simulink model "ofdm_txrx_mimo_2x"  (Simulink 6.6)
settings: Solver=VariableStepDiscrete  StartTime=0.0  StopTime=20e3  RelTol=1e-3
PreLoadFcn:
  | ofdm_rx_mimo_init
InitFcn:
  | ofdm_rx_mimo_init
StartFcn:
  | ofdm_rx_mimo_init
values from neighbouring files (symbols this model uses):
  BER_RAM_init_size = 2048    [ofdm_tx_mimo_init.m]
  CPLength = 16    [ofdm_tx_mimo_init.m]
  CRCPolynomial = hex2dec('04c11db7')    [ofdm_tx_mimo_init.m]
  freqOffset_track_filtCoef_i = hex2dec('2500')/2^32    [ofdm_rx_mimo_init.m]
  freqOffset_track_filtCoef_p = hex2dec('d0000')/2^32    [ofdm_rx_mimo_init.m]
  hi = zeros(1,length(cci))    [ofdm_rx_mimo_init.m]
  hr = zeros(1,length(ccr))    [ofdm_rx_mimo_init.m]
  max_CP_length = 16    [ofdm_tx_mimo_init.m]
  max_num_baseRateSymbols = 31    [ofdm_tx_mimo_init.m]
  max_num_subcarriers = 64    [ofdm_tx_mimo_init.m]
  max_num_trainingSymbols = 15    [ofdm_tx_mimo_init.m]
  max_numBytes = 16384    [ofdm_tx_mimo_init.m]
  max_OFDM_symbols = 2047    [ofdm_tx_mimo_init.m]
  modConstellation_qam16 = .75*[1 3 -1 -3]./3    [ofdm_tx_mimo_init.m]
  modConstellation_qam256 = 0.9375*[3 1 5 7 11 9 13 15 -3 -1 -5 -7 -11 -9 -13 -15]./15    [ofdm_tx_mimo_init.m]
  modConstellation_qam64 = 0.875*[3 1 5 7 -3 -1 -5 -7]./7    [ofdm_tx_mimo_init.m]
  modConstellation_qpsk = [1 -1]./sqrt(2)    [ofdm_tx_mimo_init.m]
  numBaseRateSymbols = 2    [ofdm_tx_mimo_init.m]
  numSubcarriers = 64    [ofdm_tx_mimo_init.m]
  numTrainingSymbols = 2    [ofdm_tx_mimo_init.m]
  pilot1_indicies = 7 + ( (64-7) * 2^16)    (= 3735559)    [ofdm_tx_mimo_init.m]
  pilot2_indicies = 21 + ( (64-21) * 2^16)    (= 2818069)    [ofdm_tx_mimo_init.m]
  pilotValue_neg = hex2dec('8000') + (2^16 * 0)    [ofdm_tx_mimo_init.m]
  pilotValue_pos = hex2dec('7FFF') + (2^16 * 0)    [ofdm_tx_mimo_init.m]
  pkt_crossCorr_thresh = 0.7    [ofdm_rx_mimo_init.m]
  pkt_energy_thresh = 0    [ofdm_rx_mimo_init.m]
  pkt_numPayloadBytes = 1400+42-6    (= 1436)    [ofdm_tx_mimo_init.m]
  pktByteNums = numHeaderBytes +  				byteIndex_numPayloadBytes(1)*2^8 +  				byteIndex_numPayloadBytes(2)*2^16 +  				byteIndex_simpleDynModMasks * 2^24    [ofdm_rx_mimo_init.m]
  PktDet_Delay = 26 + Symbol_Timing_Offset*2^7    (= 1050)    [ofdm_rx_mimo_init.m]
  preamble = 6*[0 shortsyms_10 longsyms_2]    [ofdm_tx_mimo_init.m]
  preamble_I = real(preamble)    [ofdm_tx_mimo_init.m]
  preamble_Q = imag(preamble)    [ofdm_tx_mimo_init.m]
  RAM_init_size = max_numBytes    (= 16384)    [ofdm_tx_mimo_init.m]
  reg_Interrupt_PktBuf_Control = reg_InterruptControl +  	1 * 2^16 +  	0 * 2^24    (= 65656)    [ofdm_rx_mimo_init.m]
  rx_controlBits = 1 * 2  		 + 1 * 4  		 + 0 * 8  		 + 1 * 16 * rx_SISO_Mode  		 + 1 * 32  		 + 1 * 64  		 + 0 * 128  		 + 1 * 256  		 + 0 * 512  		 + 1 * 1024  		 + 0 * 2048  		 + 1 * 4096  		 + 1 * 8192  		 + 0 * 16384  		 + 1 * 65536  		 + 1 * 131072  		 + 0 * 262144  		 + 1 * 524288    [ofdm_rx_mimo_init.m]
  Rx_PhaseNoiseTrack_K = 0.6094    [ofdm_rx_mimo_init.m]
  rx_postEq_scaling = round(rxScaling*2^11) * (1 + 2^16)    [ofdm_rx_mimo_init.m]
  RxDataScrambling_Seq = [40 198 78 63 82 173 102 245 48 111 172 115 147 230 216 93 72 65 62 2 205 242 122 90 128 83 105 97 73 10 5 252]    [ofdm_tx_mimo_init.m]
  simOnly_numSamples = length(preamble)+( (numSubcarriers+CPLength)*(numTrainingSymbols + numBaseRateSymbols + numFullRateSymbols/2) )    [ofdm_tx_mimo_init.m]
  train = [train train]    [ofdm_tx_mimo_init.m]
  tx_controlBits = (antB_preambleShift * 2^4) + (2^0 * tx_SISO_Mode) + 4*0 + 8*1    (= 73)    [ofdm_tx_mimo_init.m]
  TxDataScrambling_Seq = [40 198 78 63 82 173 102 245 48 111 172 115 147 230 216 93 72 65 62 2 205 242 122 90 128 83 105 97 73 10 5 252]    [ofdm_tx_mimo_init.m]
  txReg_symbolCounts = (2^16 * numFullRateSymbols) + (2^8 *numBaseRateSymbols ) + numTrainingSymbols    [ofdm_tx_mimo_init.m]
  TxRx_FFTScaling = bin2dec('010111') + (bin2dec('000101') * 2^6)    [ofdm_tx_mimo_init.m]
undefined symbols (not defined in the file or in its folder): AntA, AntB, BaseRate, CRC_table_gen, gcbh, intfilt, pktTiming_count_payloadStart, pktTiming_symbol_length, xlBlockLoadCallback, xlGetSimulinkPeriod
libraries: xbsIndex_r4 x3973, dspsigops x4, commchan3 x2, commrflib2 x2, dspsrcs4 x1
(part 19 of 38)

== system ofdm_txrx_mimo_2x/OFDM Rx MIMO/Long Corr 4x/C6/FSM ==
  Coef Addr Gen : xbsIndex_r4/Counter  cnt_type=Free Running  cnt_to=Inf  operation=Up  start_count=0  cnt_by_val=1  arith_type=Unsigned  n_bits=ceil(log2(length(h(1:N))))  bin_pt=0  load_pin=off  rst=on  en=off  explicit_period=off  period=1  dbl_ovrd=off  use_behavioral_HDL=off  use_rpm=on  implementation=Fabric  block_type=counter
      settings: xl_area=[2 3 0 3 0 0 0]  block_version=VER_STRING_GOES_HERE
  Constant2 : xbsIndex_r4/Constant  arith_type=Unsigned  const=0  n_bits=ceil(log2(length(h(1:N))/2))+1  bin_pt=0  explicit_period=off  period=2/N  equ=P=C  opselect=C  inp2=PCIN>>17  opr=+  inp1=P  carry=CIN  dbl_ovrd=off  block_type=constant
      settings: block_version=VER_STRING_GOES_HERE
  Constant3 : xbsIndex_r4/Constant  arith_type=Unsigned  const=length(h(1:N))-1  n_bits=ceil(log2(length(h(1:N))))  bin_pt=0  explicit_period=off  period=2/N  equ=P=C  opselect=C  inp2=PCIN>>17  opr=+  inp1=P  carry=CIN  dbl_ovrd=off  block_type=constant
      settings: block_version=VER_STRING_GOES_HERE
  Data Addr Gen : xbsIndex_r4/Counter  cnt_type=Count Limited  cnt_to=length(h(1:N))-1  operation=Up  start_count=0  cnt_by_val=1  arith_type=Unsigned  n_bits=ceil(log2(length(h(1:N))))  bin_pt=0  load_pin=off  rst=off  en=off  explicit_period=on  period=Ts_In/N  dbl_ovrd=off  use_behavioral_HDL=off  use_rpm=on  implementation=Fabric  block_type=counter
      settings: xl_area=[3 3 0 4 0 0 0]  block_version=VER_STRING_GOES_HERE
  Delay1 : xbsIndex_r4/Delay  en=off  latency=1  dbl_ovrd=off  reg_retiming=off  block_type=delay
      settings: infoedit=Hardware notes: A delay line is a chain, each link of which is an SRL16 followed by a flip-flop. If register retiming is enabled, the delay line is a chain of flip-flops.  xl_area=[2 3 0 0 0 0 0]  block_version=VER_STRING_GOES_HERE
  Gateway Out : xbsIndex_r4/Gateway Out  hdl_port=off  timing_constraint=None  locs_specified=off  LOCs={}  block_type=gatewayout
      settings: infoedit="Gateway out block.  Converts Xilinx fixed point inputs into ouputs of type Simulink integer, double, or fixed point.<P><P>Hardware notes:  In hardware these blocks become top level output ports or are discarded, depending on how they are configured."  xl_area=[0 0 0 0 0 0 0]  block_version=VER_STRING_GOES_HERE
  Gateway Out1 : xbsIndex_r4/Gateway Out  hdl_port=off  timing_constraint=None  locs_specified=off  LOCs={}  block_type=gatewayout
      settings: infoedit="Gateway out block.  Converts Xilinx fixed point inputs into ouputs of type Simulink integer, double, or fixed point.<P><P>Hardware notes:  In hardware these blocks become top level output ports or are discarded, depending on how they are configured."  xl_area=[0 0 0 0 0 0 0]  block_version=VER_STRING_GOES_HERE
  Gateway Out3 : xbsIndex_r4/Gateway Out  hdl_port=off  timing_constraint=None  locs_specified=off  LOCs={}  block_type=gatewayout
      settings: infoedit="Gateway out block.  Converts Xilinx fixed point inputs into ouputs of type Simulink integer, double, or fixed point.<P><P>Hardware notes:  In hardware these blocks become top level output ports or are discarded, depending on how they are configured."  xl_area=[0 0 0 0 0 0 0]  block_version=VER_STRING_GOES_HERE
  Long Corr Scope : Scope  NumInputPorts=3
      settings: YMin=-1~-1~-35  YMax=1~1~25  SaveName=ScopeData4
  Relational2 : xbsIndex_r4/Relational  mode=a=b  en=off  latency=1  dbl_ovrd=off  block_type=relational
      settings: xl_area=[1 1 0 2 0 0 0]  block_version=VER_STRING_GOES_HERE
  Relational3 : xbsIndex_r4/Relational  mode=a=b  en=off  latency=1  dbl_ovrd=off  block_type=relational
      settings: xl_area=[1 1 0 2 0 0 0]  block_version=VER_STRING_GOES_HERE
  bc3 : xbsIndex_r4/Convert  arith_type=Unsigned  n_bits=1  bin_pt=0  quantization=Truncate  overflow=Wrap  latency=0  dbl_ovrd=off  pipeline=off  block_type=convert
      settings: xl_area=[0 0 0 0 0 0 0]  block_version=VER_STRING_GOES_HERE
  Data Addr : Outport
  Coef Addr : Outport  Port=2
  load : Outport  Port=3
  wires:
  Gateway Out3/1 -> Long Corr Scope/3
  Gateway Out1/1 -> Long Corr Scope/2
  Gateway Out/1 -> Long Corr Scope/1
  Data Addr Gen/1 -> Gateway Out/1, Data Addr/1, Relational2/1
  Coef Addr Gen/1 -> Gateway Out1/1, Delay1/1
  Relational2/1 -> Coef Addr Gen/1
  Constant2/1 -> Relational2/2
  Delay1/1 -> Relational3/1, Coef Addr/1
  Constant3/1 -> Relational3/2
  Relational3/1 -> Gateway Out3/1, bc3/1
  bc3/1 -> load/1

== system ofdm_txrx_mimo_2x/OFDM Rx MIMO/Long Corr 4x/C7/C1 ==
  xn : Inport
  DAddr : Inport  Port=2
  CAddr : Inport  Port=3
  LD : Inport  Port=4
  Down Sample2 : xbsIndex_r4/Down Sample  sample_ratio=length(coef2)  sample_phase=First Value of Frame  en=off  latency=0  dbl_ovrd=off  block_type=dsamp
      settings: xl_area=[5 5 0 4 0 0 0]  block_version=VER_STRING_GOES_HERE
  MAC : Subsystem
  R : xbsIndex_r4/Addressable Shift Register  infer_latency=off  depth=length(coef2)  initVector=[0]  en=off  dbl_ovrd=off  use_behavioral_HDL=off  use_rpm=off  block_type=addrsr
      settings: infoedit="Delay of  configurable length.  Any element  in the delay     line   can    be   addressed   and   driven    onto   the   output     port.<P><P>Hardware  notes: Implemented  using SRL16s.     If Virtex-4, Virtex-II or Spartan-3 devices are used, multiple SRLC16s are cascaded together."  xl_area=[1 0 0 2 0 0 0]  block_version=VER_STRING_GOES_HERE
  ROM1 : xbsIndex_r4/ROM  depth=length(coef2)  initVector=coef2  distributed_mem=Distributed memory  rst=off  init_reg=0  en=off  latency=1  arith_type=Unsigned  n_bits=1  bin_pt=0  dbl_ovrd=off  optimize=Area  use_rpm=off  block_type=sprom
      settings: xl_area=[1 1 0 1 0 0 0]  block_version=VER_STRING_GOES_HERE
  yn : Outport
  xnz : Outport  Port=2
  wires:
  xn/1 -> R/1
  DAddr/1 -> R/2
  CAddr/1 -> ROM1/1
  R/1 -> xnz/1, MAC/1(a)
  MAC/1(yn) -> Down Sample2/1
  Down Sample2/1 -> yn/1
  ROM1/1 -> MAC/2(coeff)
  LD/1 -> MAC/3(ld)

== system ofdm_txrx_mimo_2x/OFDM Rx MIMO/Long Corr 4x/C7/C10 ==
  xn : Inport
  DAddr : Inport  Port=2
  CAddr : Inport  Port=3
  LD : Inport  Port=4
  Down Sample1 : xbsIndex_r4/Down Sample  sample_ratio=length(coef2)  sample_phase=First Value of Frame  en=off  latency=0  dbl_ovrd=off  block_type=dsamp
      settings: xl_area=[3 3 0 2 0 0 0]  block_version=VER_STRING_GOES_HERE
  Down Sample2 : xbsIndex_r4/Down Sample  sample_ratio=length(coef2)  sample_phase=First Value of Frame  en=off  latency=0  dbl_ovrd=off  block_type=dsamp
      settings: xl_area=[5 5 0 4 0 0 0]  block_version=VER_STRING_GOES_HERE
  MAC : Subsystem
  R : xbsIndex_r4/Addressable Shift Register  infer_latency=off  depth=length(coef2)  initVector=[0]  en=off  dbl_ovrd=off  use_behavioral_HDL=off  use_rpm=off  block_type=addrsr
      settings: infoedit="Delay of  configurable length.  Any element  in the delay     line   can    be   addressed   and   driven    onto   the   output     port.<P><P>Hardware  notes: Implemented  using SRL16s.     If Virtex-4, Virtex-II or Spartan-3 devices are used, multiple SRLC16s are cascaded together."  xl_area=[1 0 0 2 0 0 0]  block_version=VER_STRING_GOES_HERE
  ROM1 : xbsIndex_r4/ROM  depth=length(coef2)  initVector=coef2  distributed_mem=Distributed memory  rst=off  init_reg=0  en=off  latency=1  arith_type=Unsigned  n_bits=1  bin_pt=0  dbl_ovrd=off  optimize=Area  use_rpm=off  block_type=sprom
      settings: xl_area=[1 1 0 1 0 0 0]  block_version=VER_STRING_GOES_HERE
  yn : Outport
  xnz : Outport  Port=2
  wires:
  Down Sample1/1 -> R/1
  xn/1 -> Down Sample1/1
  DAddr/1 -> R/2
  CAddr/1 -> ROM1/1
  R/1 -> xnz/1, MAC/1(a)
  MAC/1(yn) -> Down Sample2/1
  Down Sample2/1 -> yn/1
  ROM1/1 -> MAC/2(coeff)
  LD/1 -> MAC/3(ld)

== system ofdm_txrx_mimo_2x/OFDM Rx MIMO/Long Corr 4x/C7/C11 ==
  xn : Inport
  DAddr : Inport  Port=2
  CAddr : Inport  Port=3
  LD : Inport  Port=4
  Down Sample1 : xbsIndex_r4/Down Sample  sample_ratio=length(coef2)  sample_phase=First Value of Frame  en=off  latency=0  dbl_ovrd=off  block_type=dsamp
      settings: xl_area=[3 3 0 2 0 0 0]  block_version=VER_STRING_GOES_HERE
  Down Sample2 : xbsIndex_r4/Down Sample  sample_ratio=length(coef2)  sample_phase=First Value of Frame  en=off  latency=0  dbl_ovrd=off  block_type=dsamp
      settings: xl_area=[5 5 0 4 0 0 0]  block_version=VER_STRING_GOES_HERE
  MAC : Subsystem
  R : xbsIndex_r4/Addressable Shift Register  infer_latency=off  depth=length(coef2)  initVector=[0]  en=off  dbl_ovrd=off  use_behavioral_HDL=off  use_rpm=off  block_type=addrsr
      settings: infoedit="Delay of  configurable length.  Any element  in the delay     line   can    be   addressed   and   driven    onto   the   output     port.<P><P>Hardware  notes: Implemented  using SRL16s.     If Virtex-4, Virtex-II or Spartan-3 devices are used, multiple SRLC16s are cascaded together."  xl_area=[1 0 0 2 0 0 0]  block_version=VER_STRING_GOES_HERE
  ROM1 : xbsIndex_r4/ROM  depth=length(coef2)  initVector=coef2  distributed_mem=Distributed memory  rst=off  init_reg=0  en=off  latency=1  arith_type=Unsigned  n_bits=1  bin_pt=0  dbl_ovrd=off  optimize=Area  use_rpm=off  block_type=sprom
      settings: xl_area=[1 1 0 1 0 0 0]  block_version=VER_STRING_GOES_HERE
  yn : Outport
  xnz : Outport  Port=2
  wires:
  Down Sample1/1 -> R/1
  xn/1 -> Down Sample1/1
  DAddr/1 -> R/2
  CAddr/1 -> ROM1/1
  R/1 -> xnz/1, MAC/1(a)
  MAC/1(yn) -> Down Sample2/1
  Down Sample2/1 -> yn/1
  ROM1/1 -> MAC/2(coeff)
  LD/1 -> MAC/3(ld)

== system ofdm_txrx_mimo_2x/OFDM Rx MIMO/Long Corr 4x/C7/C12 ==
  xn : Inport
  DAddr : Inport  Port=2
  CAddr : Inport  Port=3
  LD : Inport  Port=4
  Down Sample1 : xbsIndex_r4/Down Sample  sample_ratio=length(coef2)  sample_phase=First Value of Frame  en=off  latency=0  dbl_ovrd=off  block_type=dsamp
      settings: xl_area=[3 3 0 2 0 0 0]  block_version=VER_STRING_GOES_HERE
  Down Sample2 : xbsIndex_r4/Down Sample  sample_ratio=length(coef2)  sample_phase=First Value of Frame  en=off  latency=0  dbl_ovrd=off  block_type=dsamp
      settings: xl_area=[5 5 0 4 0 0 0]  block_version=VER_STRING_GOES_HERE
  MAC : Subsystem
  R : xbsIndex_r4/Addressable Shift Register  infer_latency=off  depth=length(coef2)  initVector=[0]  en=off  dbl_ovrd=off  use_behavioral_HDL=off  use_rpm=off  block_type=addrsr
      settings: infoedit="Delay of  configurable length.  Any element  in the delay     line   can    be   addressed   and   driven    onto   the   output     port.<P><P>Hardware  notes: Implemented  using SRL16s.     If Virtex-4, Virtex-II or Spartan-3 devices are used, multiple SRLC16s are cascaded together."  xl_area=[1 0 0 2 0 0 0]  block_version=VER_STRING_GOES_HERE
  ROM1 : xbsIndex_r4/ROM  depth=length(coef2)  initVector=coef2  distributed_mem=Distributed memory  rst=off  init_reg=0  en=off  latency=1  arith_type=Unsigned  n_bits=1  bin_pt=0  dbl_ovrd=off  optimize=Area  use_rpm=off  block_type=sprom
      settings: xl_area=[1 1 0 1 0 0 0]  block_version=VER_STRING_GOES_HERE
  yn : Outport
  xnz : Outport  Port=2
  wires:
  Down Sample1/1 -> R/1
  xn/1 -> Down Sample1/1
  DAddr/1 -> R/2
  CAddr/1 -> ROM1/1
  R/1 -> xnz/1, MAC/1(a)
  MAC/1(yn) -> Down Sample2/1
  Down Sample2/1 -> yn/1
  ROM1/1 -> MAC/2(coeff)
  LD/1 -> MAC/3(ld)

== system ofdm_txrx_mimo_2x/OFDM Rx MIMO/Long Corr 4x/C7/C13 ==
  xn : Inport
  DAddr : Inport  Port=2
  CAddr : Inport  Port=3
  LD : Inport  Port=4
  Down Sample1 : xbsIndex_r4/Down Sample  sample_ratio=length(coef2)  sample_phase=First Value of Frame  en=off  latency=0  dbl_ovrd=off  block_type=dsamp
      settings: xl_area=[3 3 0 2 0 0 0]  block_version=VER_STRING_GOES_HERE
  Down Sample2 : xbsIndex_r4/Down Sample  sample_ratio=length(coef2)  sample_phase=First Value of Frame  en=off  latency=0  dbl_ovrd=off  block_type=dsamp
      settings: xl_area=[5 5 0 4 0 0 0]  block_version=VER_STRING_GOES_HERE
  MAC : Subsystem
  R : xbsIndex_r4/Addressable Shift Register  infer_latency=off  depth=length(coef2)  initVector=[0]  en=off  dbl_ovrd=off  use_behavioral_HDL=off  use_rpm=off  block_type=addrsr
      settings: infoedit="Delay of  configurable length.  Any element  in the delay     line   can    be   addressed   and   driven    onto   the   output     port.<P><P>Hardware  notes: Implemented  using SRL16s.     If Virtex-4, Virtex-II or Spartan-3 devices are used, multiple SRLC16s are cascaded together."  xl_area=[1 0 0 2 0 0 0]  block_version=VER_STRING_GOES_HERE
  ROM1 : xbsIndex_r4/ROM  depth=length(coef2)  initVector=coef2  distributed_mem=Distributed memory  rst=off  init_reg=0  en=off  latency=1  arith_type=Unsigned  n_bits=1  bin_pt=0  dbl_ovrd=off  optimize=Area  use_rpm=off  block_type=sprom
      settings: xl_area=[1 1 0 1 0 0 0]  block_version=VER_STRING_GOES_HERE
  yn : Outport
  xnz : Outport  Port=2
  wires:
  Down Sample1/1 -> R/1
  xn/1 -> Down Sample1/1
  DAddr/1 -> R/2
  CAddr/1 -> ROM1/1
  R/1 -> xnz/1, MAC/1(a)
  MAC/1(yn) -> Down Sample2/1
  Down Sample2/1 -> yn/1
  ROM1/1 -> MAC/2(coeff)
  LD/1 -> MAC/3(ld)

== system ofdm_txrx_mimo_2x/OFDM Rx MIMO/Long Corr 4x/C7/C14 ==
  xn : Inport
  DAddr : Inport  Port=2
  CAddr : Inport  Port=3
  LD : Inport  Port=4
  Down Sample1 : xbsIndex_r4/Down Sample  sample_ratio=length(coef2)  sample_phase=First Value of Frame  en=off  latency=0  dbl_ovrd=off  block_type=dsamp
      settings: xl_area=[3 3 0 2 0 0 0]  block_version=VER_STRING_GOES_HERE
  Down Sample2 : xbsIndex_r4/Down Sample  sample_ratio=length(coef2)  sample_phase=First Value of Frame  en=off  latency=0  dbl_ovrd=off  block_type=dsamp
      settings: xl_area=[5 5 0 4 0 0 0]  block_version=VER_STRING_GOES_HERE
  MAC : Subsystem
  R : xbsIndex_r4/Addressable Shift Register  infer_latency=off  depth=length(coef2)  initVector=[0]  en=off  dbl_ovrd=off  use_behavioral_HDL=off  use_rpm=off  block_type=addrsr
      settings: infoedit="Delay of  configurable length.  Any element  in the delay     line   can    be   addressed   and   driven    onto   the   output     port.<P><P>Hardware  notes: Implemented  using SRL16s.     If Virtex-4, Virtex-II or Spartan-3 devices are used, multiple SRLC16s are cascaded together."  xl_area=[1 0 0 2 0 0 0]  block_version=VER_STRING_GOES_HERE
  ROM1 : xbsIndex_r4/ROM  depth=length(coef2)  initVector=coef2  distributed_mem=Distributed memory  rst=off  init_reg=0  en=off  latency=1  arith_type=Unsigned  n_bits=1  bin_pt=0  dbl_ovrd=off  optimize=Area  use_rpm=off  block_type=sprom
      settings: xl_area=[1 1 0 1 0 0 0]  block_version=VER_STRING_GOES_HERE
  yn : Outport
  xnz : Outport  Port=2
  wires:
  LD/1 -> MAC/3(ld)
  ROM1/1 -> MAC/2(coeff)
  Down Sample2/1 -> yn/1
  MAC/1(yn) -> Down Sample2/1
  R/1 -> MAC/1(a), xnz/1
  CAddr/1 -> ROM1/1
  DAddr/1 -> R/2
  xn/1 -> Down Sample1/1
  Down Sample1/1 -> R/1

== system ofdm_txrx_mimo_2x/OFDM Rx MIMO/Long Corr 4x/C7/C15 ==
  xn : Inport
  DAddr : Inport  Port=2
  CAddr : Inport  Port=3
  LD : Inport  Port=4
  Down Sample1 : xbsIndex_r4/Down Sample  sample_ratio=length(coef2)  sample_phase=First Value of Frame  en=off  latency=0  dbl_ovrd=off  block_type=dsamp
      settings: xl_area=[3 3 0 2 0 0 0]  block_version=VER_STRING_GOES_HERE
  Down Sample2 : xbsIndex_r4/Down Sample  sample_ratio=length(coef2)  sample_phase=First Value of Frame  en=off  latency=0  dbl_ovrd=off  block_type=dsamp
      settings: xl_area=[5 5 0 4 0 0 0]  block_version=VER_STRING_GOES_HERE
  MAC : Subsystem
  R : xbsIndex_r4/Addressable Shift Register  infer_latency=off  depth=length(coef2)  initVector=[0]  en=off  dbl_ovrd=off  use_behavioral_HDL=off  use_rpm=off  block_type=addrsr
      settings: infoedit="Delay of  configurable length.  Any element  in the delay     line   can    be   addressed   and   driven    onto   the   output     port.<P><P>Hardware  notes: Implemented  using SRL16s.     If Virtex-4, Virtex-II or Spartan-3 devices are used, multiple SRLC16s are cascaded together."  xl_area=[1 0 0 2 0 0 0]  block_version=VER_STRING_GOES_HERE
  ROM1 : xbsIndex_r4/ROM  depth=length(coef2)  initVector=coef2  distributed_mem=Distributed memory  rst=off  init_reg=0  en=off  latency=1  arith_type=Unsigned  n_bits=1  bin_pt=0  dbl_ovrd=off  optimize=Area  use_rpm=off  block_type=sprom
      settings: xl_area=[1 1 0 1 0 0 0]  block_version=VER_STRING_GOES_HERE
  yn : Outport
  xnz : Outport  Port=2
  wires:
  Down Sample1/1 -> R/1
  xn/1 -> Down Sample1/1
  DAddr/1 -> R/2
  CAddr/1 -> ROM1/1
  R/1 -> xnz/1, MAC/1(a)
  MAC/1(yn) -> Down Sample2/1
  Down Sample2/1 -> yn/1
  ROM1/1 -> MAC/2(coeff)
  LD/1 -> MAC/3(ld)

== system ofdm_txrx_mimo_2x/OFDM Rx MIMO/Long Corr 4x/C7/C16 ==
  xn : Inport
  DAddr : Inport  Port=2
  CAddr : Inport  Port=3
  LD : Inport  Port=4
  Down Sample1 : xbsIndex_r4/Down Sample  sample_ratio=length(coef2)  sample_phase=First Value of Frame  en=off  latency=0  dbl_ovrd=off  block_type=dsamp
      settings: xl_area=[3 3 0 2 0 0 0]  block_version=VER_STRING_GOES_HERE
  Down Sample2 : xbsIndex_r4/Down Sample  sample_ratio=length(coef2)  sample_phase=First Value of Frame  en=off  latency=0  dbl_ovrd=off  block_type=dsamp
      settings: xl_area=[5 5 0 4 0 0 0]  block_version=VER_STRING_GOES_HERE
  MAC : Subsystem
  R : xbsIndex_r4/Addressable Shift Register  infer_latency=off  depth=length(coef2)  initVector=[0]  en=off  dbl_ovrd=off  use_behavioral_HDL=off  use_rpm=off  block_type=addrsr
      settings: infoedit="Delay of  configurable length.  Any element  in the delay     line   can    be   addressed   and   driven    onto   the   output     port.<P><P>Hardware  notes: Implemented  using SRL16s.     If Virtex-4, Virtex-II or Spartan-3 devices are used, multiple SRLC16s are cascaded together."  xl_area=[1 0 0 2 0 0 0]  block_version=VER_STRING_GOES_HERE
  ROM1 : xbsIndex_r4/ROM  depth=length(coef2)  initVector=coef2  distributed_mem=Distributed memory  rst=off  init_reg=0  en=off  latency=1  arith_type=Unsigned  n_bits=1  bin_pt=0  dbl_ovrd=off  optimize=Area  use_rpm=off  block_type=sprom
      settings: xl_area=[1 1 0 1 0 0 0]  block_version=VER_STRING_GOES_HERE
  yn : Outport
  xnz : Outport  Port=2
  wires:
  LD/1 -> MAC/3(ld)
  ROM1/1 -> MAC/2(coeff)
  Down Sample2/1 -> yn/1
  MAC/1(yn) -> Down Sample2/1
  R/1 -> MAC/1(a), xnz/1
  CAddr/1 -> ROM1/1
  DAddr/1 -> R/2
  xn/1 -> Down Sample1/1
  Down Sample1/1 -> R/1

== system ofdm_txrx_mimo_2x/OFDM Rx MIMO/Long Corr 4x/C7/C2 ==
  xn : Inport
  DAddr : Inport  Port=2
  CAddr : Inport  Port=3
  LD : Inport  Port=4
  Down Sample1 : xbsIndex_r4/Down Sample  sample_ratio=length(coef2)  sample_phase=First Value of Frame  en=off  latency=0  dbl_ovrd=off  block_type=dsamp
      settings: xl_area=[3 3 0 2 0 0 0]  block_version=VER_STRING_GOES_HERE
  Down Sample2 : xbsIndex_r4/Down Sample  sample_ratio=length(coef2)  sample_phase=First Value of Frame  en=off  latency=0  dbl_ovrd=off  block_type=dsamp
      settings: xl_area=[5 5 0 4 0 0 0]  block_version=VER_STRING_GOES_HERE
  MAC : Subsystem
  R : xbsIndex_r4/Addressable Shift Register  infer_latency=off  depth=length(coef2)  initVector=[0]  en=off  dbl_ovrd=off  use_behavioral_HDL=off  use_rpm=off  block_type=addrsr
      settings: infoedit="Delay of  configurable length.  Any element  in the delay     line   can    be   addressed   and   driven    onto   the   output     port.<P><P>Hardware  notes: Implemented  using SRL16s.     If Virtex-4, Virtex-II or Spartan-3 devices are used, multiple SRLC16s are cascaded together."  xl_area=[1 0 0 2 0 0 0]  block_version=VER_STRING_GOES_HERE
  ROM1 : xbsIndex_r4/ROM  depth=length(coef2)  initVector=coef2  distributed_mem=Distributed memory  rst=off  init_reg=0  en=off  latency=1  arith_type=Unsigned  n_bits=1  bin_pt=0  dbl_ovrd=off  optimize=Area  use_rpm=off  block_type=sprom
      settings: xl_area=[1 1 0 1 0 0 0]  block_version=VER_STRING_GOES_HERE
  yn : Outport
  xnz : Outport  Port=2
  wires:
  Down Sample1/1 -> R/1
  xn/1 -> Down Sample1/1
  DAddr/1 -> R/2
  CAddr/1 -> ROM1/1
  R/1 -> xnz/1, MAC/1(a)
  MAC/1(yn) -> Down Sample2/1
  Down Sample2/1 -> yn/1
  ROM1/1 -> MAC/2(coeff)
  LD/1 -> MAC/3(ld)

== system ofdm_txrx_mimo_2x/OFDM Rx MIMO/Long Corr 4x/C7/C3 ==
  xn : Inport
  DAddr : Inport  Port=2
  CAddr : Inport  Port=3
  LD : Inport  Port=4
  Down Sample1 : xbsIndex_r4/Down Sample  sample_ratio=length(coef2)  sample_phase=First Value of Frame  en=off  latency=0  dbl_ovrd=off  block_type=dsamp
      settings: xl_area=[3 3 0 2 0 0 0]  block_version=VER_STRING_GOES_HERE
  Down Sample2 : xbsIndex_r4/Down Sample  sample_ratio=length(coef2)  sample_phase=First Value of Frame  en=off  latency=0  dbl_ovrd=off  block_type=dsamp
      settings: xl_area=[5 5 0 4 0 0 0]  block_version=VER_STRING_GOES_HERE
  MAC : Subsystem
  R : xbsIndex_r4/Addressable Shift Register  infer_latency=off  depth=length(coef2)  initVector=[0]  en=off  dbl_ovrd=off  use_behavioral_HDL=off  use_rpm=off  block_type=addrsr
      settings: infoedit="Delay of  configurable length.  Any element  in the delay     line   can    be   addressed   and   driven    onto   the   output     port.<P><P>Hardware  notes: Implemented  using SRL16s.     If Virtex-4, Virtex-II or Spartan-3 devices are used, multiple SRLC16s are cascaded together."  xl_area=[1 0 0 2 0 0 0]  block_version=VER_STRING_GOES_HERE
  ROM1 : xbsIndex_r4/ROM  depth=length(coef2)  initVector=coef2  distributed_mem=Distributed memory  rst=off  init_reg=0  en=off  latency=1  arith_type=Unsigned  n_bits=1  bin_pt=0  dbl_ovrd=off  optimize=Area  use_rpm=off  block_type=sprom
      settings: xl_area=[1 1 0 1 0 0 0]  block_version=VER_STRING_GOES_HERE
  yn : Outport
  xnz : Outport  Port=2
  wires:
  Down Sample1/1 -> R/1
  xn/1 -> Down Sample1/1
  DAddr/1 -> R/2
  CAddr/1 -> ROM1/1
  R/1 -> xnz/1, MAC/1(a)
  MAC/1(yn) -> Down Sample2/1
  Down Sample2/1 -> yn/1
  ROM1/1 -> MAC/2(coeff)
  LD/1 -> MAC/3(ld)

== system ofdm_txrx_mimo_2x/OFDM Rx MIMO/Long Corr 4x/C7/C4 ==
  xn : Inport
  DAddr : Inport  Port=2
  CAddr : Inport  Port=3
  LD : Inport  Port=4
  Down Sample1 : xbsIndex_r4/Down Sample  sample_ratio=length(coef2)  sample_phase=First Value of Frame  en=off  latency=0  dbl_ovrd=off  block_type=dsamp
      settings: xl_area=[3 3 0 2 0 0 0]  block_version=VER_STRING_GOES_HERE
  Down Sample2 : xbsIndex_r4/Down Sample  sample_ratio=length(coef2)  sample_phase=First Value of Frame  en=off  latency=0  dbl_ovrd=off  block_type=dsamp
      settings: xl_area=[5 5 0 4 0 0 0]  block_version=VER_STRING_GOES_HERE
  MAC : Subsystem
  R : xbsIndex_r4/Addressable Shift Register  infer_latency=off  depth=length(coef2)  initVector=[0]  en=off  dbl_ovrd=off  use_behavioral_HDL=off  use_rpm=off  block_type=addrsr
      settings: infoedit="Delay of  configurable length.  Any element  in the delay     line   can    be   addressed   and   driven    onto   the   output     port.<P><P>Hardware  notes: Implemented  using SRL16s.     If Virtex-4, Virtex-II or Spartan-3 devices are used, multiple SRLC16s are cascaded together."  xl_area=[1 0 0 2 0 0 0]  block_version=VER_STRING_GOES_HERE
  ROM1 : xbsIndex_r4/ROM  depth=length(coef2)  initVector=coef2  distributed_mem=Distributed memory  rst=off  init_reg=0  en=off  latency=1  arith_type=Unsigned  n_bits=1  bin_pt=0  dbl_ovrd=off  optimize=Area  use_rpm=off  block_type=sprom
      settings: xl_area=[1 1 0 1 0 0 0]  block_version=VER_STRING_GOES_HERE
  yn : Outport
  xnz : Outport  Port=2
  wires:
  Down Sample1/1 -> R/1
  xn/1 -> Down Sample1/1
  DAddr/1 -> R/2
  CAddr/1 -> ROM1/1
  R/1 -> xnz/1, MAC/1(a)
  MAC/1(yn) -> Down Sample2/1
  Down Sample2/1 -> yn/1
  ROM1/1 -> MAC/2(coeff)
  LD/1 -> MAC/3(ld)

== system ofdm_txrx_mimo_2x/OFDM Rx MIMO/Long Corr 4x/C7/C5 ==
  xn : Inport
  DAddr : Inport  Port=2
  CAddr : Inport  Port=3
  LD : Inport  Port=4
  Down Sample1 : xbsIndex_r4/Down Sample  sample_ratio=length(coef2)  sample_phase=First Value of Frame  en=off  latency=0  dbl_ovrd=off  block_type=dsamp
      settings: xl_area=[3 3 0 2 0 0 0]  block_version=VER_STRING_GOES_HERE
  Down Sample2 : xbsIndex_r4/Down Sample  sample_ratio=length(coef2)  sample_phase=First Value of Frame  en=off  latency=0  dbl_ovrd=off  block_type=dsamp
      settings: xl_area=[5 5 0 4 0 0 0]  block_version=VER_STRING_GOES_HERE
  MAC : Subsystem
  R : xbsIndex_r4/Addressable Shift Register  infer_latency=off  depth=length(coef2)  initVector=[0]  en=off  dbl_ovrd=off  use_behavioral_HDL=off  use_rpm=off  block_type=addrsr
      settings: infoedit="Delay of  configurable length.  Any element  in the delay     line   can    be   addressed   and   driven    onto   the   output     port.<P><P>Hardware  notes: Implemented  using SRL16s.     If Virtex-4, Virtex-II or Spartan-3 devices are used, multiple SRLC16s are cascaded together."  xl_area=[1 0 0 2 0 0 0]  block_version=VER_STRING_GOES_HERE
  ROM1 : xbsIndex_r4/ROM  depth=length(coef2)  initVector=coef2  distributed_mem=Distributed memory  rst=off  init_reg=0  en=off  latency=1  arith_type=Unsigned  n_bits=1  bin_pt=0  dbl_ovrd=off  optimize=Area  use_rpm=off  block_type=sprom
      settings: xl_area=[1 1 0 1 0 0 0]  block_version=VER_STRING_GOES_HERE
  yn : Outport
  xnz : Outport  Port=2
  wires:
  Down Sample1/1 -> R/1
  xn/1 -> Down Sample1/1
  DAddr/1 -> R/2
  CAddr/1 -> ROM1/1
  R/1 -> xnz/1, MAC/1(a)
  MAC/1(yn) -> Down Sample2/1
  Down Sample2/1 -> yn/1
  ROM1/1 -> MAC/2(coeff)
  LD/1 -> MAC/3(ld)

== system ofdm_txrx_mimo_2x/OFDM Rx MIMO/Long Corr 4x/C7/C6 ==
  xn : Inport
  DAddr : Inport  Port=2
  CAddr : Inport  Port=3
  LD : Inport  Port=4
  Down Sample1 : xbsIndex_r4/Down Sample  sample_ratio=length(coef2)  sample_phase=First Value of Frame  en=off  latency=0  dbl_ovrd=off  block_type=dsamp
      settings: xl_area=[3 3 0 2 0 0 0]  block_version=VER_STRING_GOES_HERE
  Down Sample2 : xbsIndex_r4/Down Sample  sample_ratio=length(coef2)  sample_phase=First Value of Frame  en=off  latency=0  dbl_ovrd=off  block_type=dsamp
      settings: xl_area=[5 5 0 4 0 0 0]  block_version=VER_STRING_GOES_HERE
  MAC : Subsystem
  R : xbsIndex_r4/Addressable Shift Register  infer_latency=off  depth=length(coef2)  initVector=[0]  en=off  dbl_ovrd=off  use_behavioral_HDL=off  use_rpm=off  block_type=addrsr
      settings: infoedit="Delay of  configurable length.  Any element  in the delay     line   can    be   addressed   and   driven    onto   the   output     port.<P><P>Hardware  notes: Implemented  using SRL16s.     If Virtex-4, Virtex-II or Spartan-3 devices are used, multiple SRLC16s are cascaded together."  xl_area=[1 0 0 2 0 0 0]  block_version=VER_STRING_GOES_HERE
  ROM1 : xbsIndex_r4/ROM  depth=length(coef2)  initVector=coef2  distributed_mem=Distributed memory  rst=off  init_reg=0  en=off  latency=1  arith_type=Unsigned  n_bits=1  bin_pt=0  dbl_ovrd=off  optimize=Area  use_rpm=off  block_type=sprom
      settings: xl_area=[1 1 0 1 0 0 0]  block_version=VER_STRING_GOES_HERE
  yn : Outport
  xnz : Outport  Port=2
  wires:
  Down Sample1/1 -> R/1
  xn/1 -> Down Sample1/1
  DAddr/1 -> R/2
  CAddr/1 -> ROM1/1
  R/1 -> xnz/1, MAC/1(a)
  MAC/1(yn) -> Down Sample2/1
  Down Sample2/1 -> yn/1
  ROM1/1 -> MAC/2(coeff)
  LD/1 -> MAC/3(ld)

== system ofdm_txrx_mimo_2x/OFDM Rx MIMO/Long Corr 4x/C7/C7 ==
  xn : Inport
  DAddr : Inport  Port=2
  CAddr : Inport  Port=3
  LD : Inport  Port=4
  Down Sample1 : xbsIndex_r4/Down Sample  sample_ratio=length(coef2)  sample_phase=First Value of Frame  en=off  latency=0  dbl_ovrd=off  block_type=dsamp
      settings: xl_area=[3 3 0 2 0 0 0]  block_version=VER_STRING_GOES_HERE
  Down Sample2 : xbsIndex_r4/Down Sample  sample_ratio=length(coef2)  sample_phase=First Value of Frame  en=off  latency=0  dbl_ovrd=off  block_type=dsamp
      settings: xl_area=[5 5 0 4 0 0 0]  block_version=VER_STRING_GOES_HERE
  MAC : Subsystem
  R : xbsIndex_r4/Addressable Shift Register  infer_latency=off  depth=length(coef2)  initVector=[0]  en=off  dbl_ovrd=off  use_behavioral_HDL=off  use_rpm=off  block_type=addrsr
      settings: infoedit="Delay of  configurable length.  Any element  in the delay     line   can    be   addressed   and   driven    onto   the   output     port.<P><P>Hardware  notes: Implemented  using SRL16s.     If Virtex-4, Virtex-II or Spartan-3 devices are used, multiple SRLC16s are cascaded together."  xl_area=[1 0 0 2 0 0 0]  block_version=VER_STRING_GOES_HERE
  ROM1 : xbsIndex_r4/ROM  depth=length(coef2)  initVector=coef2  distributed_mem=Distributed memory  rst=off  init_reg=0  en=off  latency=1  arith_type=Unsigned  n_bits=1  bin_pt=0  dbl_ovrd=off  optimize=Area  use_rpm=off  block_type=sprom
      settings: xl_area=[1 1 0 1 0 0 0]  block_version=VER_STRING_GOES_HERE
  yn : Outport
  xnz : Outport  Port=2
  wires:
  Down Sample1/1 -> R/1
  xn/1 -> Down Sample1/1
  DAddr/1 -> R/2
  CAddr/1 -> ROM1/1
  R/1 -> xnz/1, MAC/1(a)
  MAC/1(yn) -> Down Sample2/1
  Down Sample2/1 -> yn/1
  ROM1/1 -> MAC/2(coeff)
  LD/1 -> MAC/3(ld)

== system ofdm_txrx_mimo_2x/OFDM Rx MIMO/Long Corr 4x/C7/C8 ==
  xn : Inport
  DAddr : Inport  Port=2
  CAddr : Inport  Port=3
  LD : Inport  Port=4
  Down Sample1 : xbsIndex_r4/Down Sample  sample_ratio=length(coef2)  sample_phase=First Value of Frame  en=off  latency=0  dbl_ovrd=off  block_type=dsamp
      settings: xl_area=[3 3 0 2 0 0 0]  block_version=VER_STRING_GOES_HERE
  Down Sample2 : xbsIndex_r4/Down Sample  sample_ratio=length(coef2)  sample_phase=First Value of Frame  en=off  latency=0  dbl_ovrd=off  block_type=dsamp
      settings: xl_area=[5 5 0 4 0 0 0]  block_version=VER_STRING_GOES_HERE
  MAC : Subsystem
  R : xbsIndex_r4/Addressable Shift Register  infer_latency=off  depth=length(coef2)  initVector=[0]  en=off  dbl_ovrd=off  use_behavioral_HDL=off  use_rpm=off  block_type=addrsr
      settings: infoedit="Delay of  configurable length.  Any element  in the delay     line   can    be   addressed   and   driven    onto   the   output     port.<P><P>Hardware  notes: Implemented  using SRL16s.     If Virtex-4, Virtex-II or Spartan-3 devices are used, multiple SRLC16s are cascaded together."  xl_area=[1 0 0 2 0 0 0]  block_version=VER_STRING_GOES_HERE
  ROM1 : xbsIndex_r4/ROM  depth=length(coef2)  initVector=coef2  distributed_mem=Distributed memory  rst=off  init_reg=0  en=off  latency=1  arith_type=Unsigned  n_bits=1  bin_pt=0  dbl_ovrd=off  optimize=Area  use_rpm=off  block_type=sprom
      settings: xl_area=[1 1 0 1 0 0 0]  block_version=VER_STRING_GOES_HERE
  yn : Outport
  xnz : Outport  Port=2
  wires:
  Down Sample1/1 -> R/1
  xn/1 -> Down Sample1/1
  DAddr/1 -> R/2
  CAddr/1 -> ROM1/1
  R/1 -> xnz/1, MAC/1(a)
  MAC/1(yn) -> Down Sample2/1
  Down Sample2/1 -> yn/1
  ROM1/1 -> MAC/2(coeff)
  LD/1 -> MAC/3(ld)

== system ofdm_txrx_mimo_2x/OFDM Rx MIMO/Long Corr 4x/C7/C9 ==
  xn : Inport
  DAddr : Inport  Port=2
  CAddr : Inport  Port=3
  LD : Inport  Port=4
  Down Sample1 : xbsIndex_r4/Down Sample  sample_ratio=length(coef2)  sample_phase=First Value of Frame  en=off  latency=0  dbl_ovrd=off  block_type=dsamp
      settings: xl_area=[3 3 0 2 0 0 0]  block_version=VER_STRING_GOES_HERE
  Down Sample2 : xbsIndex_r4/Down Sample  sample_ratio=length(coef2)  sample_phase=First Value of Frame  en=off  latency=0  dbl_ovrd=off  block_type=dsamp
      settings: xl_area=[5 5 0 4 0 0 0]  block_version=VER_STRING_GOES_HERE
  MAC : Subsystem
  R : xbsIndex_r4/Addressable Shift Register  infer_latency=off  depth=length(coef2)  initVector=[0]  en=off  dbl_ovrd=off  use_behavioral_HDL=off  use_rpm=off  block_type=addrsr
      settings: infoedit="Delay of  configurable length.  Any element  in the delay     line   can    be   addressed   and   driven    onto   the   output     port.<P><P>Hardware  notes: Implemented  using SRL16s.     If Virtex-4, Virtex-II or Spartan-3 devices are used, multiple SRLC16s are cascaded together."  xl_area=[1 0 0 2 0 0 0]  block_version=VER_STRING_GOES_HERE
  ROM1 : xbsIndex_r4/ROM  depth=length(coef2)  initVector=coef2  distributed_mem=Distributed memory  rst=off  init_reg=0  en=off  latency=1  arith_type=Unsigned  n_bits=1  bin_pt=0  dbl_ovrd=off  optimize=Area  use_rpm=off  block_type=sprom
      settings: xl_area=[1 1 0 1 0 0 0]  block_version=VER_STRING_GOES_HERE
  yn : Outport
  xnz : Outport  Port=2
  wires:
  Down Sample1/1 -> R/1
  xn/1 -> Down Sample1/1
  DAddr/1 -> R/2
  CAddr/1 -> ROM1/1
  R/1 -> xnz/1, MAC/1(a)
  MAC/1(yn) -> Down Sample2/1
  Down Sample2/1 -> yn/1
  ROM1/1 -> MAC/2(coeff)
  LD/1 -> MAC/3(ld)

== system ofdm_txrx_mimo_2x/OFDM Rx MIMO/Long Corr 4x/C7/FSM ==
  Coef Addr Gen : xbsIndex_r4/Counter  cnt_type=Free Running  cnt_to=Inf  operation=Up  start_count=0  cnt_by_val=1  arith_type=Unsigned  n_bits=ceil(log2(length(h(1:N))))  bin_pt=0  load_pin=off  rst=on  en=off  explicit_period=off  period=1  dbl_ovrd=off  use_behavioral_HDL=off  use_rpm=on  implementation=Fabric  block_type=counter
      settings: xl_area=[2 3 0 3 0 0 0]  block_version=VER_STRING_GOES_HERE
  Constant2 : xbsIndex_r4/Constant  arith_type=Unsigned  const=0  n_bits=ceil(log2(length(h(1:N))/2))+1  bin_pt=0  explicit_period=off  period=2/N  equ=P=C  opselect=C  inp2=PCIN>>17  opr=+  inp1=P  carry=CIN  dbl_ovrd=off  block_type=constant
      settings: block_version=VER_STRING_GOES_HERE
  Constant3 : xbsIndex_r4/Constant  arith_type=Unsigned  const=length(h(1:N))-1  n_bits=ceil(log2(length(h(1:N))))  bin_pt=0  explicit_period=off  period=2/N  equ=P=C  opselect=C  inp2=PCIN>>17  opr=+  inp1=P  carry=CIN  dbl_ovrd=off  block_type=constant
      settings: block_version=VER_STRING_GOES_HERE
  Data Addr Gen : xbsIndex_r4/Counter  cnt_type=Count Limited  cnt_to=length(h(1:N))-1  operation=Up  start_count=0  cnt_by_val=1  arith_type=Unsigned  n_bits=ceil(log2(length(h(1:N))))  bin_pt=0  load_pin=off  rst=off  en=off  explicit_period=on  period=Ts_In/N  dbl_ovrd=off  use_behavioral_HDL=off  use_rpm=on  implementation=Fabric  block_type=counter
      settings: xl_area=[3 3 0 4 0 0 0]  block_version=VER_STRING_GOES_HERE
  Delay1 : xbsIndex_r4/Delay  en=off  latency=1  dbl_ovrd=off  reg_retiming=off  block_type=delay
      settings: infoedit=Hardware notes: A delay line is a chain, each link of which is an SRL16 followed by a flip-flop. If register retiming is enabled, the delay line is a chain of flip-flops.  xl_area=[2 3 0 0 0 0 0]  block_version=VER_STRING_GOES_HERE
  Gateway Out : xbsIndex_r4/Gateway Out  hdl_port=off  timing_constraint=None  locs_specified=off  LOCs={}  block_type=gatewayout
      settings: infoedit="Gateway out block.  Converts Xilinx fixed point inputs into ouputs of type Simulink integer, double, or fixed point.<P><P>Hardware notes:  In hardware these blocks become top level output ports or are discarded, depending on how they are configured."  xl_area=[0 0 0 0 0 0 0]  block_version=VER_STRING_GOES_HERE
  Gateway Out1 : xbsIndex_r4/Gateway Out  hdl_port=off  timing_constraint=None  locs_specified=off  LOCs={}  block_type=gatewayout
      settings: infoedit="Gateway out block.  Converts Xilinx fixed point inputs into ouputs of type Simulink integer, double, or fixed point.<P><P>Hardware notes:  In hardware these blocks become top level output ports or are discarded, depending on how they are configured."  xl_area=[0 0 0 0 0 0 0]  block_version=VER_STRING_GOES_HERE
  Gateway Out3 : xbsIndex_r4/Gateway Out  hdl_port=off  timing_constraint=None  locs_specified=off  LOCs={}  block_type=gatewayout
      settings: infoedit="Gateway out block.  Converts Xilinx fixed point inputs into ouputs of type Simulink integer, double, or fixed point.<P><P>Hardware notes:  In hardware these blocks become top level output ports or are discarded, depending on how they are configured."  xl_area=[0 0 0 0 0 0 0]  block_version=VER_STRING_GOES_HERE
  Long Corr Scope : Scope  NumInputPorts=3
      settings: YMin=-1~-1~-35  YMax=1~1~25  SaveName=ScopeData4
  Relational2 : xbsIndex_r4/Relational  mode=a=b  en=off  latency=1  dbl_ovrd=off  block_type=relational
      settings: xl_area=[1 1 0 2 0 0 0]  block_version=VER_STRING_GOES_HERE
  Relational3 : xbsIndex_r4/Relational  mode=a=b  en=off  latency=1  dbl_ovrd=off  block_type=relational
      settings: xl_area=[1 1 0 2 0 0 0]  block_version=VER_STRING_GOES_HERE
  bc3 : xbsIndex_r4/Convert  arith_type=Unsigned  n_bits=1  bin_pt=0  quantization=Truncate  overflow=Wrap  latency=0  dbl_ovrd=off  pipeline=off  block_type=convert
      settings: xl_area=[0 0 0 0 0 0 0]  block_version=VER_STRING_GOES_HERE
  Data Addr : Outport
  Coef Addr : Outport  Port=2
  load : Outport  Port=3
  wires:
  bc3/1 -> load/1
  Relational3/1 -> bc3/1, Gateway Out3/1
  Constant3/1 -> Relational3/2
  Delay1/1 -> Coef Addr/1, Relational3/1
  Constant2/1 -> Relational2/2
  Relational2/1 -> Coef Addr Gen/1
  Coef Addr Gen/1 -> Delay1/1, Gateway Out1/1
  Data Addr Gen/1 -> Relational2/1, Data Addr/1, Gateway Out/1
  Gateway Out/1 -> Long Corr Scope/1
  Gateway Out1/1 -> Long Corr Scope/2
  Gateway Out3/1 -> Long Corr Scope/3

== system ofdm_txrx_mimo_2x/OFDM Rx MIMO/Packet_Timing_Control/Disable for Interrupts/S-R Latch ==
  S : Inport
  R : Inport  Port=2
  Constant1 : xbsIndex_r4/Constant  arith_type=Boolean  const=1  n_bits=1  bin_pt=0  explicit_period=off  period=1  equ=P=C  opselect=C  inp2=PCIN>>17  opr=+  inp1=P  carry=CIN  dbl_ovrd=off  block_type=constant
      settings: block_version=VER_STRING_GOES_HERE
  Register2 : xbsIndex_r4/Register  init=0  rst=on  en=on  dbl_ovrd=off  block_type=register
      settings: xl_area=[1 1 0 0 0 0 0]  block_version=VER_STRING_GOES_HERE
  Q : Outport
  wires:
  Constant1/1 -> Register2/1
  R/1 -> Register2/2
  Register2/1 -> Q/1
  S/1 -> Register2/3

== system ofdm_txrx_mimo_2x/OFDM Tx MIMO/OutputBuffers/Addr Gen/1 Shift Reg ==
  d : Inport
  rst : Inport  Port=2
  en : Inport  Port=3
  Payload Running1 : xbsIndex_r4/Register  init=0  rst=on  en=on  dbl_ovrd=off  block_type=register
      settings: xl_area=[1 1 0 0 0 0 0]  block_version=VER_STRING_GOES_HERE
  Q : Outport
  wires:
  en/1 -> Payload Running1/3
  rst/1 -> Payload Running1/2
  d/1 -> Payload Running1/1
  Payload Running1/1 -> Q/1

== system ofdm_txrx_mimo_2x/OFDM Tx MIMO/OutputBuffers/Addr Gen/1 Shift Reg1 ==
  d : Inport
  rst : Inport  Port=2
  en : Inport  Port=3
  Payload Running1 : xbsIndex_r4/Register  init=1  rst=on  en=on  dbl_ovrd=off  block_type=register
      settings: xl_area=[1 1 0 0 0 0 0]  block_version=VER_STRING_GOES_HERE
  Q : Outport
  wires:
  Payload Running1/1 -> Q/1
  d/1 -> Payload Running1/1
  rst/1 -> Payload Running1/2
  en/1 -> Payload Running1/3

== system ofdm_txrx_mimo_2x/OFDM Tx MIMO/OutputBuffers/Addr Gen/2 Shift Reg ==
  d : Inport
  en : Inport  Port=2
  rst : Inport  Port=3
  Payload Running1 : xbsIndex_r4/Register  init=0  rst=on  en=on  dbl_ovrd=off  block_type=register
      settings: xl_area=[1 1 0 0 0 0 0]  block_version=VER_STRING_GOES_HERE
  Payload Running3 : xbsIndex_r4/Register  init=0  rst=on  en=on  dbl_ovrd=off  block_type=register
      settings: xl_area=[1 1 0 0 0 0 0]  block_version=VER_STRING_GOES_HERE
  Q : Outport
  wires:
  en/1 -> Payload Running1/3, Payload Running3/3
  rst/1 -> Payload Running1/2, Payload Running3/2
  d/1 -> Payload Running1/1
  Payload Running3/1 -> Q/1
  Payload Running1/1 -> Payload Running3/1

== system ofdm_txrx_mimo_2x/OFDM Tx MIMO/OutputBuffers/Addr Gen/Oscillator ==
  period : Inport
  rst : Inport  Port=2
  en : Inport  Port=3
  Assert : xbsIndex_r4/Assert  Orientation=left  assert_type=on  type_source=Explicitly  arith_type=Boolean  n_bits=16  bin_pt=14  assert_rate=on  rate_source=Explicitly  period=2  output_port=on  block_type=assert
      settings: infoedit=Asserts a user-defined sample rate and/or type on a signal.<P><P>Hardware notes: In hardware this block costs nothing.  block_version=VER_STRING_GOES_HERE
  Inverter1 : xbsIndex_r4/Inverter  Orientation=left  en=off  latency=0  dbl_ovrd=off  block_type=inv
      settings: block_version=VER_STRING_GOES_HERE
  Mux : xbsIndex_r4/Mux  inputs=2  en=off  latency=0  precision=Full  arith_type=Unsigned  n_bits=16  bin_pt=14  quantization=Truncate  overflow=Wrap  dbl_ovrd=off  block_type=mux
      settings: block_version=VER_STRING_GOES_HERE
  Register : xbsIndex_r4/Register  init=0  rst=on  en=on  dbl_ovrd=off  block_type=register
      settings: block_version=VER_STRING_GOES_HERE
  Register1 : xbsIndex_r4/Register  init=0  rst=on  en=on  dbl_ovrd=off  block_type=register
      settings: block_version=VER_STRING_GOES_HERE
  Q : Outport
  wires:
  rst/1 -> Register/2, Register1/2
  Assert/1 -> Inverter1/1
  Inverter1/1 -> Register/1, Mux/3
  en/1 -> Register/3, Register1/3
  Register1/1 -> Q/1, Assert/1
  Mux/1 -> Register1/1
  period/1 -> Mux/1
  Register/1 -> Mux/2

== system ofdm_txrx_mimo_2x/OFDM Tx MIMO/OutputBuffers/Addr Gen/Oscillator1 ==
  en : Inport
  rst : Inport  Port=2
  Assert : xbsIndex_r4/Assert  Orientation=left  assert_type=on  type_source=Explicitly  arith_type=Boolean  n_bits=16  bin_pt=14  assert_rate=on  rate_source=Explicitly  period=2  output_port=on  block_type=assert
      settings: infoedit=Asserts a user-defined sample rate and/or type on a signal.<P><P>Hardware notes: In hardware this block costs nothing.  block_version=VER_STRING_GOES_HERE
  Inverter1 : xbsIndex_r4/Inverter  Orientation=left  en=off  latency=0  dbl_ovrd=off  block_type=inv
      settings: block_version=VER_STRING_GOES_HERE
  Register : xbsIndex_r4/Register  init=0  rst=on  en=on  dbl_ovrd=off  block_type=register
      settings: block_version=VER_STRING_GOES_HERE
  Q : Outport
  wires:
  Register/1 -> Assert/1, Q/1
  en/1 -> Register/3
  Inverter1/1 -> Register/1
  Assert/1 -> Inverter1/1
  rst/1 -> Register/2

== system ofdm_txrx_mimo_2x/OFDM Tx MIMO/OutputBuffers/Addr Gen/Rd Counter ==
  En : Inport
  Rst : Inport  Port=2
  6LSB : xbsIndex_r4/Slice  nbits=log2(numSubcarriers)  boolean_output=off  mode=Lower Bit Location + Width  bit1=0  base1=MSB of Input  bit0=0  base0=LSB of Input  dbl_ovrd=off  block_type=slice
      settings: infoedit="Extracts a given range of bits from each input sample and presents it at the output.  The output type is ordinarily unsigned with binary point at zero, but can be Boolean when the slice is one bit wide.<P><P>Hardware notes: In hardware this block costs nothing."  block_version=VER_STRING_GOES_HERE
  Constant3 : xbsIndex_r4/Constant  Orientation=left  arith_type=Unsigned  const=numSubcarriers + CPLength - 1  n_bits=ceil(log2(numSubcarriers + CPLength - 1))  bin_pt=0  explicit_period=off  period=2  equ=P=C  opselect=C  inp2=PCIN>>17  opr=+  inp1=P  carry=CIN  dbl_ovrd=off  block_type=constant
      settings: block_version=VER_STRING_GOES_HERE
  Logical : xbsIndex_r4/Logical  Orientation=up  logical_function=OR  inputs=2  en=off  latency=0  precision=Full  arith_type=Unsigned  n_bits=16  bin_pt=0  align_bp=on  dbl_ovrd=off  block_type=logical
      settings: block_version=VER_STRING_GOES_HERE
  Read Counter : xbsIndex_r4/Counter  cnt_type=Free Running  cnt_to=numSubcarriers + CPLength - 1  operation=Up  start_count=0  cnt_by_val=1  arith_type=Unsigned  n_bits=ceil(log2(numSubcarriers + CPLength - 1))  bin_pt=0  load_pin=off  rst=on  en=on  explicit_period=off  period=2  dbl_ovrd=off  use_behavioral_HDL=off  use_rpm=off  implementation=Fabric  block_type=counter
      settings: block_version=VER_STRING_GOES_HERE
  Relational : xbsIndex_r4/Relational  Orientation=left  mode=a=b  en=off  latency=0  dbl_ovrd=off  block_type=relational
      settings: block_version=VER_STRING_GOES_HERE
  Q : Outport
  Ovfl : Outport  Port=2
  wires:
  Read Counter/1 -> 6LSB/1, Relational/1
  Relational/1 -> Logical/2, Ovfl/1
  Logical/1 -> Read Counter/1
  Constant3/1 -> Relational/2
  En/1 -> Read Counter/2
  Rst/1 -> Logical/1
  6LSB/1 -> Q/1

== system ofdm_txrx_mimo_2x/OFDM Tx MIMO/OutputBuffers/Addr Gen/SR Latch ==
  S : Inport
  R : Inport  Port=2
  Constant1 : xbsIndex_r4/Constant  arith_type=Boolean  const=1  n_bits=1  bin_pt=0  explicit_period=off  period=2  equ=P=C  opselect=C  inp2=PCIN>>17  opr=+  inp1=P  carry=CIN  dbl_ovrd=off  block_type=constant
      settings: block_version=VER_STRING_GOES_HERE
  Payload Running3 : xbsIndex_r4/Register  init=0  rst=on  en=on  dbl_ovrd=off  block_type=register
      settings: xl_area=[1 1 0 0 0 0 0]  block_version=VER_STRING_GOES_HERE
  Q : Outport
  wires:
  Constant1/1 -> Payload Running3/1
  R/1 -> Payload Running3/2
  S/1 -> Payload Running3/3
  Payload Running3/1 -> Q/1
